simulink model "CCMLoad"  (R2022b)
settings: Solver=FixedStepDiscrete  StartTime=0.0  StopTime=10.0  FixedStep=0.005  RelTol=1e-3
model workspace (MAT data in the model file):
  NvMBlockCarCfgBulkData = Simulink.Signal
  NvMBlockCarCfgBulkData.DataType = 'UInt8'
  NvMBlockCarCfgBulkData.Dimensions = [62 1]
  NvMBlockCarCfgBulkData.DimensionsMode = 'auto'
  NvMBlockCarCfgBulkData.Complexity = 'real'
  NvMBlockCarCfgBulkData.SampleTime = -1
  NvMBlockCarCfgBulkData.SamplingMode = 'auto'
  NvMBlockCarCfgBulkData.InitialValue = 'NvMBlockCarCfgBulkDataInit'
  NvMBlockCarCfgBulkDataInit = Simulink.Parameter
  NvMBlockCarCfgBulkDataInit.Value = [255; 255; 255; 255; 255; 255; 255; 255; 255; 255; 255; 255; 255; 255; 255; 255; 255; 255; 255; 255; 255; 255; 255; 255; 255; 255; 255; 255; 255; 255; 255; 255; 255; 255; 255; 255; 255; 255; 255; 255; 255; 255; 255; 255; 255; 255; 255; 255; 255; 255; 255; 255; 255; 255; 255; 255; 255; 255; 255; 255; 255; 255]
  NvMBlockCarCfgBulkDataInit.DataType = 'UInt8'
  NvMBlockCarCfgBulkDataInit.Complexity = 'real'
  NvMBlockCarCfgBulkDataInit.Dimensions = [62 1]
undefined symbols (not defined in the file or in its folder): DioChannelType, IcuChannelType, NvMBlockId, P_DioLevelType, P_Std_ReturnType, P_u16DutyCycle, PwmChannelType
(part 1 of 2)
settings Model: Creator=blue.ge  LastModifiedBy=blue.ge
settings DataIOCC: SaveFormat=StructureWithTime
settings OptimizationCC: DefaultParameterBehavior=Inlined  EnhancedBackFolding=on  PassReuseOutputArgsAs=Individual arguments  ZeroExternalMemoryAtStartup=off  ZeroInternalMemoryAtStartup=off  OptimizeBlockOrder=speed  DifferentSizesBufferReuse=on  OptimizationCustomize=off  EfficientTunableParamExpr=on
settings DebuggingCC: ParameterTunabilityLossMsg=error
settings ModelReferenceCC: ModelReferenceNumInstancesAllowed=Single
settings SFSimCC: MATLABDynamicMemAlloc=off
settings RTWCC: Description=AUTOSAR  SystemTargetFile=autosar.tlc  GenCodeOnly=on  TemplateMakefile=ert_default_tmf
settings CodeAppCC: ForceParamTrailComments=on  IgnoreCustomStorageClasses=off  OperatorAnnotations=on  SimulinkDataObjDesc=on  SFDataObjDesc=on  InsertBlockDesc=on
settings STFCustomTargetCC: TargetLangStandard=C99 (ISO)  MultiwordLength=256  ParMdlRefBuildCompliant=on  CompOptLevelCompliant=on  IncludeMdlTerminateFcn=off  CombineOutputUpdateFcns=on  SuppressErrorStatus=on  ERTCustomFileBanners=on  SupportNonFinite=off  SupportComplex=off  SupportContinuousTime=off  SupportNonInlinedSFcns=off  RemoveResetFunc=on  MATLABClassNameForMDSCustomization=Simulink.SoftwareTarget.ERTCustomization  AutosarCompliant=on  UseToolchainInfoCompliant=on  LookupTableObjectStructAxisOrder=2,1,3,4,...  LUTObjectStructOrderEvenSpacing=Size,Table,Breakpoints  SystemTargetFile=autosar.tlc
settings ERTTargetCC: TargetLangStandard=C99 (ISO)  ConcurrentExecutionCompliant=off  IncludeMdlTerminateFcn=off  SuppressErrorStatus=on  SupportNonFinite=off  SupportComplex=off  ModelStepFunctionPrototypeControlCompliant=off  CPPClassGenCompliant=off  AutosarCompliant=on  LookupTableObjectStructAxisOrder=2,1,3,4,...  LUTObjectStructOrderEvenSpacing=Size,Table,Breakpoints  InlinedParameterPlacement=NonHierarchical  ERTCustomFileTemplate=example_file_process.tlc

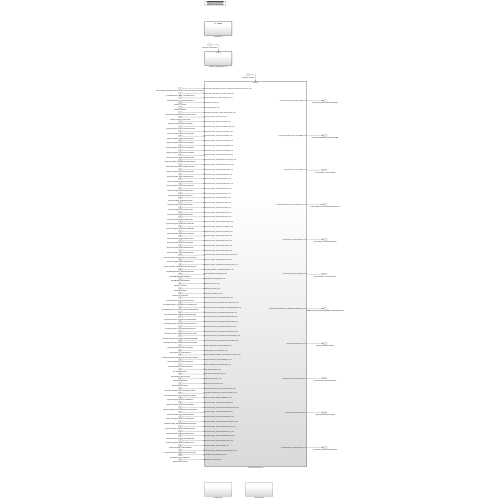
[thumbnail: Simulink's stored preview of the top-level diagram]
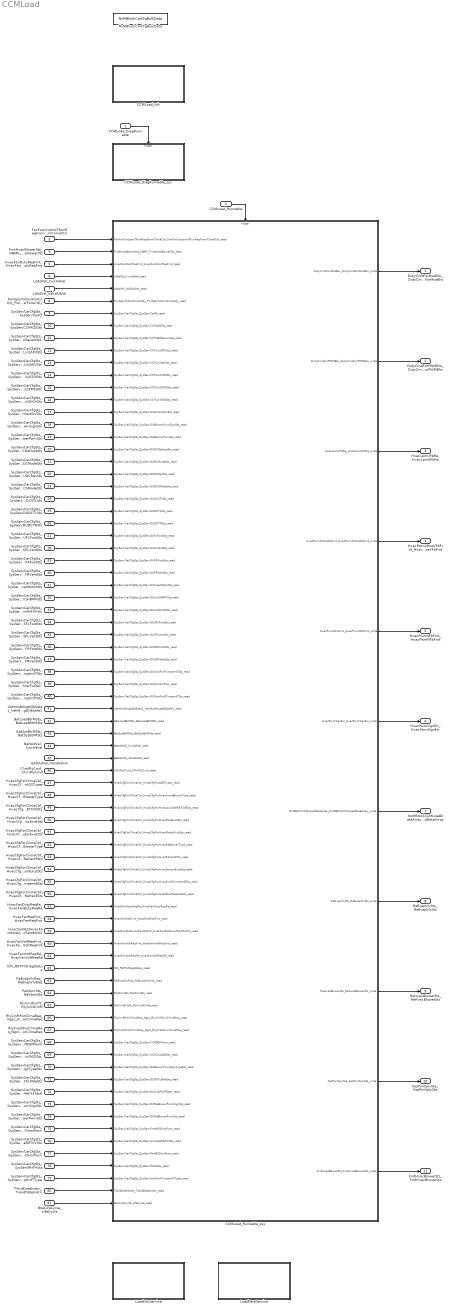
== system CCMLoad ==
  CCMLoad_DiagRunnable : Inport  SampleTime=0.005  OutputFunctionCall=on
  CCMLoad_Runnable : Inport  Port=2  SampleTime=0.02  OutputFunctionCall=on
  FanForCmptmtTRunReqFromClimaCtrl_FanForCmptmtTRunReqFromClimaCtrl : Inport  Port=3  OutDataTypeStr=Enum: OnOff1  PortDimensions=1
  FrntHvacBlowerSts_HBMF_FrntHvacBlowerSts : Inport  Port=4  OutDataTypeStr=Enum: HvacFanSts  PortDimensions=1
  HvacFanDutyReqFrnt_HvacFanDutyReqFrnt : Inport  Port=5  OutMin=0  OutMax=100  OutDataTypeStr=Perc9  PortDimensions=1
  LdAdVal_CurrAdVal : Inport  Port=6  OutDataTypeStr=Bus: LdAd  PortDimensions=1  BusOutputAsStruct=on
  LdAdVal_ValidAdVal : Inport  Port=7  OutDataTypeStr=Bus: LdAd  PortDimensions=1  BusOutputAsStruct=on
  PwrSplyToSnsrForAirQly_PwrSplyToSnsrForAirQly : Inport  Port=8  OutDataTypeStr=Enum: OnOff1  PortDimensions=1
  SysServCarCfgSts_SysServCarID : Inport  Port=9  OutDataTypeStr=Enum: SysServCarID  PortDimensions=1
  SysServCarCfgSts_SysServCCPAQSSts : Inport  Port=10  OutDataTypeStr=UInt8  PortDimensions=1
  SysServCarCfgSts_SysServCCPHEXSensorSts : Inport  Port=11  OutDataTypeStr=UInt8  PortDimensions=1
  SysServCarCfgSts_SysServCCPLin2AFUSts : Inport  Port=12  OutDataTypeStr=Boolean  PortDimensions=1
  SysServCarCfgSts_SysServCCPLin2AIUSts : Inport  Port=13  OutDataTypeStr=UInt8  PortDimensions=1
  SysServCarCfgSts_SysServCCPLin2CDSSts : Inport  Port=14  OutDataTypeStr=Boolean  PortDimensions=1
  SysServCarCfgSts_SysServCCPLin2PMSISts : Inport  Port=15  OutDataTypeStr=Boolean  PortDimensions=1
  SysServCarCfgSts_SysServCCPLin3VGASts : Inport  Port=16  OutDataTypeStr=Boolean  PortDimensions=1
  SysServCarCfgSts_SysServDUAirVentSnrSts : Inport  Port=17  OutDataTypeStr=Boolean  PortDimensions=1
  SysServCarCfgSts_SysServDUBlowerPwmSignSts : Inport  Port=18  OutDataTypeStr=Boolean  PortDimensions=1
  SysServCarCfgSts_SysServDUBlowerPwmSts : Inport  Port=19  OutDataTypeStr=Boolean  PortDimensions=1
  SysServCarCfgSts_SysServDUDCDefrostSts : Inport  Port=20  OutDataTypeStr=UInt8  PortDimensions=1
  SysServCarCfgSts_SysServDUDCModeSts : Inport  Port=21  OutDataTypeStr=UInt8  PortDimensions=1
  SysServCarCfgSts_SysServDUDCRecSts : Inport  Port=22  OutDataTypeStr=UInt8  PortDimensions=1
  SysServCarCfgSts_SysServDUDCSModeSts : Inport  Port=23  OutDataTypeStr=UInt8  PortDimensions=1
  SysServCarCfgSts_SysServDUDCSTLSts : Inport  Port=24  OutDataTypeStr=UInt8  PortDimensions=1
  SysServCarCfgSts_SysServDUDCTLSts : Inport  Port=25  OutDataTypeStr=UInt8  PortDimensions=1
  SysServCarCfgSts_SysServDUDCTRSts : Inport  Port=26  OutDataTypeStr=UInt8  PortDimensions=1
  SysServCarCfgSts_SysServDUFLFootSts : Inport  Port=27  OutDataTypeStr=UInt8  PortDimensions=1
  SysServCarCfgSts_SysServDUFLVentSts : Inport  Port=28  OutDataTypeStr=UInt8  PortDimensions=1
  SysServCarCfgSts_SysServDUFRFootSts : Inport  Port=29  OutDataTypeStr=UInt8  PortDimensions=1
  SysServCarCfgSts_SysServDUFRVentSts : Inport  Port=30  OutDataTypeStr=UInt8  PortDimensions=1
  SysServCarCfgSts_SysServDUIncarMotorSts : Inport  Port=31  OutDataTypeStr=Boolean  PortDimensions=1
  SysServCarCfgSts_SysServDULin1HBMFSts : Inport  Port=32  OutDataTypeStr=Boolean  PortDimensions=1
  SysServCarCfgSts_SysServDULin4HFAFSts : Inport  Port=33  OutDataTypeStr=UInt32  PortDimensions=1
  SysServCarCfgSts_SysServDUSFLFootSts : Inport  Port=34  OutDataTypeStr=UInt8  PortDimensions=1
  SysServCarCfgSts_SysServDUSFLVentSts : Inport  Port=35  OutDataTypeStr=UInt8  PortDimensions=1
  SysServCarCfgSts_SysServDUSFRFootSts : Inport  Port=36  OutDataTypeStr=UInt8  PortDimensions=1
  SysServCarCfgSts_SysServDUSFRVentSts : Inport  Port=37  OutDataTypeStr=UInt8  PortDimensions=1
  SysServCarCfgSts_SysServDUSnsrForFCmptmtTSts : Inport  Port=38  OutDataTypeStr=UInt8  PortDimensions=1
  SysServCarCfgSts_SysServDUSnsrForRair : Inport  Port=39  OutDataTypeStr=UInt8  PortDimensions=1
  SysServCarCfgSts_SysServDUSnsrForRCmptmtTSts : Inport  Port=40  OutDataTypeStr=UInt8  PortDimensions=1
  VehModMngtGlbSafe1_VehModMngtGlbSafe1 : Inport  Port=41  OutDataTypeStr=Bus: VehModMngtGlbSafe1  PortDimensions=1  BusOutputAsStruct=on
  BatLoadBtrMSts_BatLoadBtrMSts : Inport  Port=42  OutDataTypeStr=Enum: BatLoadBtrMStsEnum  PortDimensions=1
  BatSysBtrMSts_BatSysBtrMSts : Inport  Port=43  OutDataTypeStr=Enum: BatBtrMStsEnum  PortDimensions=1
  BattAdVal_CurrAdVal : Inport  Port=44  OutDataTypeStr=BatCurrAd  PortDimensions=1
  BattAdVal_ValidAdVal : Inport  Port=45  OutDataTypeStr=BatValidAd  PortDimensions=1
  ClimRlyCmd_ClimRlyCmd : Inport  Port=46  OutDataTypeStr=Enum: OnOff1  PortDimensions=1
  HvacCfgForClimaCtrl_HvacCfgForAQSTypes : Inport  Port=47  OutDataTypeStr=UInt8  PortDimensions=1
  HvacCfgForClimaCtrl_HvacCfgForHvacFrontBlowerType : Inport  Port=48  OutDataTypeStr=HvacCfgForHvacFrontBlowerType  PortDimensions=1
  HvacCfgForClimaCtrl_HvacCfgForHvacLowSideRETXVESts : Inport  Port=49  OutDataTypeStr=UInt8  PortDimensions=1
  HvacCfgForClimaCtrl_HvacCfgForHvacMiscExistSts : Inport  Port=50  OutDataTypeStr=HvacCfgForHvacMiscExistSts  PortDimensions=1
  HvacCfgForClimaCtrl_HvacCfgForHvacMotorExistSts : Inport  Port=51  OutDataTypeStr=HvacCfgForHvacMotorExistSts  PortDimensions=1
  HvacCfgForClimaCtrl_HvacCfgForHvacReBlowerType : Inport  Port=52  OutDataTypeStr=UInt8  PortDimensions=1
  HvacCfgForClimaCtrl_HvacCfgForHvacReVentPstn : Inport  Port=53  OutDataTypeStr=UInt8  PortDimensions=1
  HvacCfgForClimaCtrl_HvacCfgForHvacSensorExistSts : Inport  Port=54  OutDataTypeStr=HvacCfgForHvacSensorExistSts  PortDimensions=1
  HvacCfgForClimaCtrl_HvacCfgForHvacSnsrRCmptmtESts : Inport  Port=55  OutDataTypeStr=UInt8  PortDimensions=1
  HvacCfgForClimaCtrl_HvacCfgForHvacSnsrReHexESts : Inport  Port=56  OutDataTypeStr=UInt8  PortDimensions=1
  HvacFanDutyReqRe_HvacFanDutyReqRe : Inport  Port=57  OutMin=0  OutMax=100  OutDataTypeStr=Perc9  PortDimensions=1
  HvacFanReqFrnt_HvacFanReqFrnt : Inport  Port=58  OutDataTypeStr=HvacFanSpd  PortDimensions=1
  HvacFanSts2HvacFanMotU2_HvacFanSts2HvacFanMotU2 : Inport  Port=59  OutMin=0  OutMax=25.400000000000002  OutDataTypeStr=U8  PortDimensions=1
  HvacFanVoltReqFrnt_HvacFanVoltReqFrnt : Inport  Port=60  OutMin=0  OutMax=100  OutDataTypeStr=Perc9  PortDimensions=1
  HvacFanVoltReqRe_HvacFanVoltReqRe : Inport  Port=61  OutMin=0  OutMax=100  OutDataTypeStr=Perc9  PortDimensions=1
  IDX_RETXVDiagStatus : Inport  Port=62  OutDataTypeStr=UInt8  PortDimensions=1
  ReEvapVlvReq_ReEvapVlvReq : Inport  Port=63  OutDataTypeStr=Enum: OnOff1  PortDimensions=1
  ReVbmrSts_ReVbmrSts : Inport  Port=64  OutDataTypeStr=Enum: HvacFanSts  PortDimensions=1
  RlyCmdCmft_RlyCmdCmft : Inport  Port=65  OutDataTypeStr=Enum: OnOff1  PortDimensions=1
  RlyCmftForClimaReq_Ngcc_RlyCmftForClimaReq : Inport  Port=66  OutDataTypeStr=Enum: OnOff1  PortDimensions=1
  RlyCrashForClimaReq_Ngcc_RlyCrashForClimaReq : Inport  Port=67  OutDataTypeStr=Enum: OnOff1  PortDimensions=1
  SysServCarCfgSts_SysServCCPBGMNum : Inport  Port=68  OutDataTypeStr=UInt8  PortDimensions=1
  SysServCarCfgSts_SysServCCPLin2AQSSts : Inport  Port=69  OutDataTypeStr=Boolean  PortDimensions=1
  SysServCarCfgSts_SysServDUBlowerPwmSignTypeSts : Inport  Port=70  OutDataTypeStr=UInt8  PortDimensions=1
  SysServCarCfgSts_SysServDUDCFLMdeSts : Inport  Port=71  OutDataTypeStr=UInt8  PortDimensions=1
  SysServCarCfgSts_SysServDULin4HFAFStsH : Inport  Port=72  OutDataTypeStr=UInt32  PortDimensions=1
  SysServCarCfgSts_SysServDUReBlowerPwmSignSts : Inport  Port=73  OutDataTypeStr=UInt8  PortDimensions=1
  SysServCarCfgSts_SysServDUReBlowerPwmSts : Inport  Port=74  OutDataTypeStr=UInt8  PortDimensions=1
  SysServCarCfgSts_SysServFrntHEXSnsrPosn : Inport  Port=75  OutDataTypeStr=UInt8  PortDimensions=1
  SysServCarCfgSts_SysServLowSideRETXVSts : Inport  Port=76  OutDataTypeStr=UInt8  PortDimensions=1
  SysServCarCfgSts_SysServReHEXSnsrPosn : Inport  Port=77  OutDataTypeStr=UInt8  PortDimensions=1
  SysServCarCfgSts_SysServRHFASts : Inport  Port=78  OutDataTypeStr=UInt8  PortDimensions=1
  SysServCarCfgSts_SysServSnsrForFCmptmtTType : Inport  Port=79  OutDataTypeStr=UInt8  PortDimensions=1
  TiAndDateIndcn_TiAndDateIndcn : Inport  Port=80  OutDataTypeStr=Bus: DateTi30  PortDimensions=1  BusOutputAsStruct=on
  BswLifeCycle_LifeCycle : Inport  Port=81  OutDataTypeStr=Enum: CCMLifeCycleModeGroup  PortDimensions=1
  CCMLoad_DiagRunnable_sys : Subsystem
  CCMLoad_Init : Subsystem
  CCMLoad_Runnable_sys : Subsystem
  LoadDtcService : Subsystem
  LoadParaService : Subsystem
  NvMBlockCarCfgBulkData : DataStoreMemory  DataStoreName=NvMBlockCarCfgBulkData  InitialValue=NvMBlockCarCfgBulkDataInit  ShowAdditionalParam=off  Dimensions=62  StateMustResolveToSignalObject=on
  DutyCmdForMosBlw_DutyCmdForMosBlw : Outport  OutMin=0  OutMax=100  OutDataTypeStr=Perc9  PortDimensions=1
  DutyCmdForPWMBlw_DutyCmdForPWMBlw : Outport  Port=2  OutMin=0  OutMax=100  OutDataTypeStr=Perc9  PortDimensions=1
  HvacLpmUFbRe_HvacLpmUFbRe : Outport  Port=3  OutMin=0  OutMax=100  OutDataTypeStr=Perc9  PortDimensions=1
  HvacPwm2MosVFbFrnt_HvacPwm2MosVFbFrnt : Outport  Port=4  OutMin=0  OutMax=100  OutDataTypeStr=Perc9  PortDimensions=1
  HvacPwmNFbFrnt_HvacPwmNFbFrnt : Outport  Port=5  OutDataTypeStr=HvacFanSpd  PortDimensions=1
  HvacPwmSignErr_HvacPwmSignErr : Outport  Port=6  OutDataTypeStr=UInt8  PortDimensions=1
  NvMBlockCCMLoadDataArray_NvMBlockCCMLoadDataArray : Outport  Port=7  OutDataTypeStr=UInt8  PortDimensions=62
  ReEvapVlvSts_ReEvapVlvSts : Outport  Port=8  OutDataTypeStr=Enum: EvaprVlvSts  PortDimensions=1
  ReHvacBlowerSts_ReHvacBlowerSts : Outport  Port=9  OutDataTypeStr=Enum: HvacFanSts  PortDimensions=1
  AqsPwrSplySta_AqsPwrSplySta : Outport  Port=10  OutDataTypeStr=AqsPwrSplySta  PortDimensions=1
  FrntHvacBlowerSts_FrntHvacBlowerSts : Outport  Port=11  OutDataTypeStr=Enum: HvacFanSts  PortDimensions=1
  wires:
  FanForCmptmtTRunReqFromClimaCtrl_FanForCmptmtTRunReqFromClimaCtrl/1 -> CCMLoad_Runnable_sys/1(FanForCmptmtTRunReqFromClimaCtrl_FanForCmptmtTRunReqFromClimaCtrl_read)
  FrntHvacBlowerSts_HBMF_FrntHvacBlowerSts/1 -> CCMLoad_Runnable_sys/2(FrntHvacBlowerSts_HBMF_FrntHvacBlowerSts_read)
  HvacFanDutyReqFrnt_HvacFanDutyReqFrnt/1 -> CCMLoad_Runnable_sys/3(HvacFanDutyReqFrnt_HvacFanDutyReqFrnt_read)
  LdAdVal_CurrAdVal/1 -> CCMLoad_Runnable_sys/4(LdAdVal_CurrAdVal_read)
  LdAdVal_ValidAdVal/1 -> CCMLoad_Runnable_sys/5(LdAdVal_ValidAdVal_read)
  PwrSplyToSnsrForAirQly_PwrSplyToSnsrForAirQly/1 -> CCMLoad_Runnable_sys/6(PwrSplyToSnsrForAirQly_PwrSplyToSnsrForAirQly_read)
  SysServCarCfgSts_SysServCarID/1 -> CCMLoad_Runnable_sys/7(SysServCarCfgSts_SysServCarID_read)
  SysServCarCfgSts_SysServCCPAQSSts/1 -> CCMLoad_Runnable_sys/8(SysServCarCfgSts_SysServCCPAQSSts_read)
  SysServCarCfgSts_SysServCCPHEXSensorSts/1 -> CCMLoad_Runnable_sys/9(SysServCarCfgSts_SysServCCPHEXSensorSts_read)
  SysServCarCfgSts_SysServCCPLin2AFUSts/1 -> CCMLoad_Runnable_sys/10(SysServCarCfgSts_SysServCCPLin2AFUSts_read)
  SysServCarCfgSts_SysServCCPLin2AIUSts/1 -> CCMLoad_Runnable_sys/11(SysServCarCfgSts_SysServCCPLin2AIUSts_read)
  SysServCarCfgSts_SysServCCPLin2CDSSts/1 -> CCMLoad_Runnable_sys/12(SysServCarCfgSts_SysServCCPLin2CDSSts_read)
  SysServCarCfgSts_SysServCCPLin2PMSISts/1 -> CCMLoad_Runnable_sys/13(SysServCarCfgSts_SysServCCPLin2PMSISts_read)
  SysServCarCfgSts_SysServCCPLin3VGASts/1 -> CCMLoad_Runnable_sys/14(SysServCarCfgSts_SysServCCPLin3VGASts_read)
  SysServCarCfgSts_SysServDUAirVentSnrSts/1 -> CCMLoad_Runnable_sys/15(SysServCarCfgSts_SysServDUAirVentSnrSts_read)
  SysServCarCfgSts_SysServDUBlowerPwmSignSts/1 -> CCMLoad_Runnable_sys/16(SysServCarCfgSts_SysServDUBlowerPwmSignSts_read)
  SysServCarCfgSts_SysServDUBlowerPwmSts/1 -> CCMLoad_Runnable_sys/17(SysServCarCfgSts_SysServDUBlowerPwmSts_read)
  SysServCarCfgSts_SysServDUDCDefrostSts/1 -> CCMLoad_Runnable_sys/18(SysServCarCfgSts_SysServDUDCDefrostSts_read)
  SysServCarCfgSts_SysServDUDCModeSts/1 -> CCMLoad_Runnable_sys/19(SysServCarCfgSts_SysServDUDCModeSts_read)
  SysServCarCfgSts_SysServDUDCRecSts/1 -> CCMLoad_Runnable_sys/20(SysServCarCfgSts_SysServDUDCRecSts_read)
  SysServCarCfgSts_SysServDUDCSModeSts/1 -> CCMLoad_Runnable_sys/21(SysServCarCfgSts_SysServDUDCSModeSts_read)
  SysServCarCfgSts_SysServDUDCSTLSts/1 -> CCMLoad_Runnable_sys/22(SysServCarCfgSts_SysServDUDCSTLSts_read)
  SysServCarCfgSts_SysServDUDCTLSts/1 -> CCMLoad_Runnable_sys/23(SysServCarCfgSts_SysServDUDCTLSts_read)
  SysServCarCfgSts_SysServDUDCTRSts/1 -> CCMLoad_Runnable_sys/24(SysServCarCfgSts_SysServDUDCTRSts_read)
  SysServCarCfgSts_SysServDUFLFootSts/1 -> CCMLoad_Runnable_sys/25(SysServCarCfgSts_SysServDUFLFootSts_read)
  SysServCarCfgSts_SysServDUFLVentSts/1 -> CCMLoad_Runnable_sys/26(SysServCarCfgSts_SysServDUFLVentSts_read)
  SysServCarCfgSts_SysServDUFRFootSts/1 -> CCMLoad_Runnable_sys/27(SysServCarCfgSts_SysServDUFRFootSts_read)
  SysServCarCfgSts_SysServDUFRVentSts/1 -> CCMLoad_Runnable_sys/28(SysServCarCfgSts_SysServDUFRVentSts_read)
  SysServCarCfgSts_SysServDUIncarMotorSts/1 -> CCMLoad_Runnable_sys/29(SysServCarCfgSts_SysServDUIncarMotorSts_read)
  SysServCarCfgSts_SysServDULin1HBMFSts/1 -> CCMLoad_Runnable_sys/30(SysServCarCfgSts_SysServDULin1HBMFSts_read)
  SysServCarCfgSts_SysServDULin4HFAFSts/1 -> CCMLoad_Runnable_sys/31(SysServCarCfgSts_SysServDULin4HFAFSts_read)
  SysServCarCfgSts_SysServDUSFLFootSts/1 -> CCMLoad_Runnable_sys/32(SysServCarCfgSts_SysServDUSFLFootSts_read)
  SysServCarCfgSts_SysServDUSFLVentSts/1 -> CCMLoad_Runnable_sys/33(SysServCarCfgSts_SysServDUSFLVentSts_read)
  SysServCarCfgSts_SysServDUSFRFootSts/1 -> CCMLoad_Runnable_sys/34(SysServCarCfgSts_SysServDUSFRFootSts_read)
  SysServCarCfgSts_SysServDUSFRVentSts/1 -> CCMLoad_Runnable_sys/35(SysServCarCfgSts_SysServDUSFRVentSts_read)
  SysServCarCfgSts_SysServDUSnsrForFCmptmtTSts/1 -> CCMLoad_Runnable_sys/36(SysServCarCfgSts_SysServDUSnsrForFCmptmtTSts_read)
  SysServCarCfgSts_SysServDUSnsrForRair/1 -> CCMLoad_Runnable_sys/37(SysServCarCfgSts_SysServDUSnsrForRair_read)
  SysServCarCfgSts_SysServDUSnsrForRCmptmtTSts/1 -> CCMLoad_Runnable_sys/38(SysServCarCfgSts_SysServDUSnsrForRCmptmtTSts_read)
  VehModMngtGlbSafe1_VehModMngtGlbSafe1/1 -> CCMLoad_Runnable_sys/39(VehModMngtGlbSafe1_VehModMngtGlbSafe1_read)
  BatLoadBtrMSts_BatLoadBtrMSts/1 -> CCMLoad_Runnable_sys/40(BatLoadBtrMSts_BatLoadBtrMSts_read)
  BatSysBtrMSts_BatSysBtrMSts/1 -> CCMLoad_Runnable_sys/41(BatSysBtrMSts_BatSysBtrMSts_read)
  BattAdVal_CurrAdVal/1 -> CCMLoad_Runnable_sys/42(BattAdVal_CurrAdVal_read)
  BattAdVal_ValidAdVal/1 -> CCMLoad_Runnable_sys/43(BattAdVal_ValidAdVal_read)
  ClimRlyCmd_ClimRlyCmd/1 -> CCMLoad_Runnable_sys/44(ClimRlyCmd_ClimRlyCmd_read)
  HvacCfgForClimaCtrl_HvacCfgForAQSTypes/1 -> CCMLoad_Runnable_sys/45(HvacCfgForClimaCtrl_HvacCfgForAQSTypes_read)
  HvacCfgForClimaCtrl_HvacCfgForHvacFrontBlowerType/1 -> CCMLoad_Runnable_sys/46(HvacCfgForClimaCtrl_HvacCfgForHvacFrontBlowerType_read)
  HvacCfgForClimaCtrl_HvacCfgForHvacLowSideRETXVESts/1 -> CCMLoad_Runnable_sys/47(HvacCfgForClimaCtrl_HvacCfgForHvacLowSideRETXVESts_read)
  HvacCfgForClimaCtrl_HvacCfgForHvacMiscExistSts/1 -> CCMLoad_Runnable_sys/48(HvacCfgForClimaCtrl_HvacCfgForHvacMiscExistSts_read)
  HvacCfgForClimaCtrl_HvacCfgForHvacMotorExistSts/1 -> CCMLoad_Runnable_sys/49(HvacCfgForClimaCtrl_HvacCfgForHvacMotorExistSts_read)
  HvacCfgForClimaCtrl_HvacCfgForHvacReBlowerType/1 -> CCMLoad_Runnable_sys/50(HvacCfgForClimaCtrl_HvacCfgForHvacReBlowerType_read)
  HvacCfgForClimaCtrl_HvacCfgForHvacReVentPstn/1 -> CCMLoad_Runnable_sys/51(HvacCfgForClimaCtrl_HvacCfgForHvacReVentPstn_read)
  HvacCfgForClimaCtrl_HvacCfgForHvacSensorExistSts/1 -> CCMLoad_Runnable_sys/52(HvacCfgForClimaCtrl_HvacCfgForHvacSensorExistSts_read)
  HvacCfgForClimaCtrl_HvacCfgForHvacSnsrRCmptmtESts/1 -> CCMLoad_Runnable_sys/53(HvacCfgForClimaCtrl_HvacCfgForHvacSnsrRCmptmtESts_read)
  HvacCfgForClimaCtrl_HvacCfgForHvacSnsrReHexESts/1 -> CCMLoad_Runnable_sys/54(HvacCfgForClimaCtrl_HvacCfgForHvacSnsrReHexESts_read)
  HvacFanDutyReqRe_HvacFanDutyReqRe/1 -> CCMLoad_Runnable_sys/55(HvacFanDutyReqRe_HvacFanDutyReqRe_read)
  HvacFanReqFrnt_HvacFanReqFrnt/1 -> CCMLoad_Runnable_sys/56(HvacFanReqFrnt_HvacFanReqFrnt_read)
  HvacFanSts2HvacFanMotU2_HvacFanSts2HvacFanMotU2/1 -> CCMLoad_Runnable_sys/57(HvacFanSts2HvacFanMotU2_HvacFanSts2HvacFanMotU2_read)
  HvacFanVoltReqFrnt_HvacFanVoltReqFrnt/1 -> CCMLoad_Runnable_sys/58(HvacFanVoltReqFrnt_HvacFanVoltReqFrnt_read)
  HvacFanVoltReqRe_HvacFanVoltReqRe/1 -> CCMLoad_Runnable_sys/59(HvacFanVoltReqRe_HvacFanVoltReqRe_read)
  IDX_RETXVDiagStatus/1 -> CCMLoad_Runnable_sys/60(IDX_RETXVDiagStatus_read)
  ReEvapVlvReq_ReEvapVlvReq/1 -> CCMLoad_Runnable_sys/61(ReEvapVlvReq_ReEvapVlvReq_read)
  ReVbmrSts_ReVbmrSts/1 -> CCMLoad_Runnable_sys/62(ReVbmrSts_ReVbmrSts_read)
  RlyCmdCmft_RlyCmdCmft/1 -> CCMLoad_Runnable_sys/63(RlyCmdCmft_RlyCmdCmft_read)
  RlyCmftForClimaReq_Ngcc_RlyCmftForClimaReq/1 -> CCMLoad_Runnable_sys/64(RlyCmftForClimaReq_Ngcc_RlyCmftForClimaReq_read)
  RlyCrashForClimaReq_Ngcc_RlyCrashForClimaReq/1 -> CCMLoad_Runnable_sys/65(RlyCrashForClimaReq_Ngcc_RlyCrashForClimaReq_read)
  SysServCarCfgSts_SysServCCPBGMNum/1 -> CCMLoad_Runnable_sys/66(SysServCarCfgSts_SysServCCPBGMNum_read)
  SysServCarCfgSts_SysServCCPLin2AQSSts/1 -> CCMLoad_Runnable_sys/67(SysServCarCfgSts_SysServCCPLin2AQSSts_read)
  SysServCarCfgSts_SysServDUBlowerPwmSignTypeSts/1 -> CCMLoad_Runnable_sys/68(SysServCarCfgSts_SysServDUBlowerPwmSignTypeSts_read)
  SysServCarCfgSts_SysServDUDCFLMdeSts/1 -> CCMLoad_Runnable_sys/69(SysServCarCfgSts_SysServDUDCFLMdeSts_read)
  SysServCarCfgSts_SysServDULin4HFAFStsH/1 -> CCMLoad_Runnable_sys/70(SysServCarCfgSts_SysServDULin4HFAFStsH_read)
  SysServCarCfgSts_SysServDUReBlowerPwmSignSts/1 -> CCMLoad_Runnable_sys/71(SysServCarCfgSts_SysServDUReBlowerPwmSignSts_read)
  SysServCarCfgSts_SysServDUReBlowerPwmSts/1 -> CCMLoad_Runnable_sys/72(SysServCarCfgSts_SysServDUReBlowerPwmSts_read)
  SysServCarCfgSts_SysServFrntHEXSnsrPosn/1 -> CCMLoad_Runnable_sys/73(SysServCarCfgSts_SysServFrntHEXSnsrPosn_read)
  SysServCarCfgSts_SysServLowSideRETXVSts/1 -> CCMLoad_Runnable_sys/74(SysServCarCfgSts_SysServLowSideRETXVSts_read)
  SysServCarCfgSts_SysServReHEXSnsrPosn/1 -> CCMLoad_Runnable_sys/75(SysServCarCfgSts_SysServReHEXSnsrPosn_read)
  SysServCarCfgSts_SysServRHFASts/1 -> CCMLoad_Runnable_sys/76(SysServCarCfgSts_SysServRHFASts_read)
  SysServCarCfgSts_SysServSnsrForFCmptmtTType/1 -> CCMLoad_Runnable_sys/77(SysServCarCfgSts_SysServSnsrForFCmptmtTType_read)
  TiAndDateIndcn_TiAndDateIndcn/1 -> CCMLoad_Runnable_sys/78(TiAndDateIndcn_TiAndDateIndcn_read)
  CCMLoad_Runnable_sys/1(DutyCmdForMosBlw_DutyCmdForMosBlw_write) -> DutyCmdForMosBlw_DutyCmdForMosBlw/1
  CCMLoad_Runnable_sys/2(DutyCmdForPWMBlw_DutyCmdForPWMBlw_write) -> DutyCmdForPWMBlw_DutyCmdForPWMBlw/1
  CCMLoad_Runnable_sys/3(HvacLpmUFbRe_HvacLpmUFbRe_write) -> HvacLpmUFbRe_HvacLpmUFbRe/1
  CCMLoad_Runnable_sys/4(HvacPwm2MosVFbFrnt_HvacPwm2MosVFbFrnt_write) -> HvacPwm2MosVFbFrnt_HvacPwm2MosVFbFrnt/1
  CCMLoad_Runnable_sys/5(HvacPwmNFbFrnt_HvacPwmNFbFrnt_write) -> HvacPwmNFbFrnt_HvacPwmNFbFrnt/1
  CCMLoad_Runnable_sys/6(HvacPwmSignErr_HvacPwmSignErr_write) -> HvacPwmSignErr_HvacPwmSignErr/1
  CCMLoad_Runnable_sys/7(NvMBlockCCMLoadDataArray_NvMBlockCCMLoadDataArray_write) -> NvMBlockCCMLoadDataArray_NvMBlockCCMLoadDataArray/1
  CCMLoad_Runnable_sys/8(ReEvapVlvSts_ReEvapVlvSts_write) -> ReEvapVlvSts_ReEvapVlvSts/1
  CCMLoad_Runnable_sys/9(ReHvacBlowerSts_ReHvacBlowerSts_write) -> ReHvacBlowerSts_ReHvacBlowerSts/1
  CCMLoad_Runnable_sys/10(AqsPwrSplySta_AqsPwrSplySta_write) -> AqsPwrSplySta_AqsPwrSplySta/1
  CCMLoad_Runnable_sys/11(FrntHvacBlowerSts_FrntHvacBlowerSts_write) -> FrntHvacBlowerSts_FrntHvacBlowerSts/1
  BswLifeCycle_LifeCycle/1 -> CCMLoad_Runnable_sys/79(BswLifeCycle_LifeCycle_read)
  CCMLoad_DiagRunnable/1 -> CCMLoad_DiagRunnable_sys/Trigger
  CCMLoad_Runnable/1 -> CCMLoad_Runnable_sys/Trigger

== system CCMLoad/CCMLoad_DiagRunnable_sys ==
  function : TriggerPort  TriggerType=function-call  VariantControl=(inherit)  SampleTimeType=periodic  SampleTime=-1
  PA_NvMBlockCarCfgBulkDataInit : Constant  Value=NvMBlockCarCfgBulkDataInit
  Terminator_1 : Terminator
  wires:
  PA_NvMBlockCarCfgBulkDataInit/1 -> Terminator_1/1

== system CCMLoad/CCMLoad_Init ==
  Event Listener : EventListener  EventName=reset
  PA_NvMBlockCarCfgBulkDataInit : Constant  Value=NvMBlockCarCfgBulkDataInit
  Terminator_1 : Terminator
  wires:
  PA_NvMBlockCarCfgBulkDataInit/1 -> Terminator_1/1

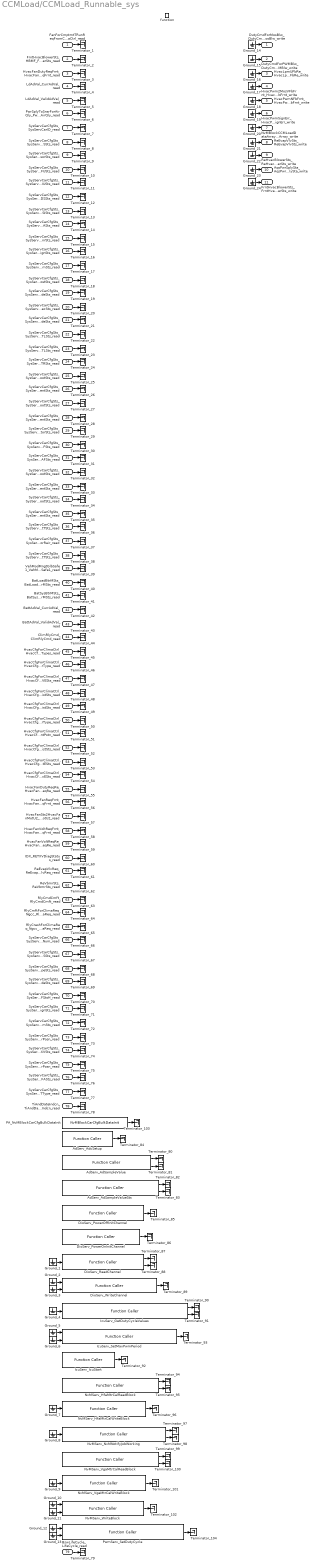
== system CCMLoad/CCMLoad_Runnable_sys ==
  FanForCmptmtTRunReqFromClimaCtrl_FanForCmptmtTRunReqFromClimaCtrl_read : Inport
  FrntHvacBlowerSts_HBMF_FrntHvacBlowerSts_read : Inport  Port=2
  HvacFanDutyReqFrnt_HvacFanDutyReqFrnt_read : Inport  Port=3
  LdAdVal_CurrAdVal_read : Inport  Port=4
  LdAdVal_ValidAdVal_read : Inport  Port=5
  PwrSplyToSnsrForAirQly_PwrSplyToSnsrForAirQly_read : Inport  Port=6
  SysServCarCfgSts_SysServCarID_read : Inport  Port=7
  SysServCarCfgSts_SysServCCPAQSSts_read : Inport  Port=8
  SysServCarCfgSts_SysServCCPHEXSensorSts_read : Inport  Port=9
  SysServCarCfgSts_SysServCCPLin2AFUSts_read : Inport  Port=10
  SysServCarCfgSts_SysServCCPLin2AIUSts_read : Inport  Port=11
  SysServCarCfgSts_SysServCCPLin2CDSSts_read : Inport  Port=12
  SysServCarCfgSts_SysServCCPLin2PMSISts_read : Inport  Port=13
  SysServCarCfgSts_SysServCCPLin3VGASts_read : Inport  Port=14
  SysServCarCfgSts_SysServDUAirVentSnrSts_read : Inport  Port=15
  SysServCarCfgSts_SysServDUBlowerPwmSignSts_read : Inport  Port=16
  SysServCarCfgSts_SysServDUBlowerPwmSts_read : Inport  Port=17
  SysServCarCfgSts_SysServDUDCDefrostSts_read : Inport  Port=18
  SysServCarCfgSts_SysServDUDCModeSts_read : Inport  Port=19
  SysServCarCfgSts_SysServDUDCRecSts_read : Inport  Port=20
  SysServCarCfgSts_SysServDUDCSModeSts_read : Inport  Port=21
  SysServCarCfgSts_SysServDUDCSTLSts_read : Inport  Port=22
  SysServCarCfgSts_SysServDUDCTLSts_read : Inport  Port=23
  SysServCarCfgSts_SysServDUDCTRSts_read : Inport  Port=24
  SysServCarCfgSts_SysServDUFLFootSts_read : Inport  Port=25
  SysServCarCfgSts_SysServDUFLVentSts_read : Inport  Port=26
  SysServCarCfgSts_SysServDUFRFootSts_read : Inport  Port=27
  SysServCarCfgSts_SysServDUFRVentSts_read : Inport  Port=28
  SysServCarCfgSts_SysServDUIncarMotorSts_read : Inport  Port=29
  SysServCarCfgSts_SysServDULin1HBMFSts_read : Inport  Port=30
  SysServCarCfgSts_SysServDULin4HFAFSts_read : Inport  Port=31
  SysServCarCfgSts_SysServDUSFLFootSts_read : Inport  Port=32
  SysServCarCfgSts_SysServDUSFLVentSts_read : Inport  Port=33
  SysServCarCfgSts_SysServDUSFRFootSts_read : Inport  Port=34
  SysServCarCfgSts_SysServDUSFRVentSts_read : Inport  Port=35
  SysServCarCfgSts_SysServDUSnsrForFCmptmtTSts_read : Inport  Port=36
  SysServCarCfgSts_SysServDUSnsrForRair_read : Inport  Port=37
  SysServCarCfgSts_SysServDUSnsrForRCmptmtTSts_read : Inport  Port=38
  VehModMngtGlbSafe1_VehModMngtGlbSafe1_read : Inport  Port=39
  BatLoadBtrMSts_BatLoadBtrMSts_read : Inport  Port=40
  BatSysBtrMSts_BatSysBtrMSts_read : Inport  Port=41
  BattAdVal_CurrAdVal_read : Inport  Port=42
  BattAdVal_ValidAdVal_read : Inport  Port=43
  ClimRlyCmd_ClimRlyCmd_read : Inport  Port=44
  HvacCfgForClimaCtrl_HvacCfgForAQSTypes_read : Inport  Port=45
  HvacCfgForClimaCtrl_HvacCfgForHvacFrontBlowerType_read : Inport  Port=46
  HvacCfgForClimaCtrl_HvacCfgForHvacLowSideRETXVESts_read : Inport  Port=47
  HvacCfgForClimaCtrl_HvacCfgForHvacMiscExistSts_read : Inport  Port=48
  HvacCfgForClimaCtrl_HvacCfgForHvacMotorExistSts_read : Inport  Port=49